simulink model "Demo_IntelligentTrafficSigns_cs"  (Simulink 7.6)
settings: Solver=FixedStepDiscrete  SolverMode=SingleTasking  StartTime=0.0  StopTime=66  FixedStep=1/10  RelTol=1e-3
PreLoadFcn:
  | check_NUTS_defined
  | 
  | assignin('base',['model_closed_' bdroot],false);
InitFcn:
  | PreScanExperimentInitFcn(bdroot)
StopFcn:
  | PreScanExperimentStopFcn(bdroot);
  | 
undefined symbols (not defined in the file or in its folder): DiscIntNeverNeededParam, magnitude, permission, R_curve, Results, Results_20090205_104801, spare_10, spare_4, spare_5, spare_6, spare_7, spare_8, spare_9, velocity_limit, x_ini_VRML, y_ini_VRML, z_ini_VRML
libraries: simulink_extras x8, PreScanSimulinkLibrary x7, simulink x5, PreScanUsefulBlocks x3
(part 9 of 10)

== system Demo_IntelligentTrafficSigns_cs/VISUWheels_BMW_X5_1/conv_RotWheelRR ==
  BryantISO [deg] : Inport
  Fcn10 : Fcn  Expr=cos(u(1))*sin(u(3))+sin(u(1))*sin(u(2))*cos(u(3))
  Fcn11 : Fcn  Expr=sin(u(1))*sin(u(3))-cos(u(1))*sin(u(2))*cos(u(3))
  Fcn12 : Fcn  Expr=-cos(u(2))*sin(u(3))
  Fcn13 : Fcn  Expr=cos(u(1))*cos(u(3))-sin(u(1))*sin(u(2))*sin(u(3))
  Fcn14 : Fcn  Expr=sin(u(1))*cos(u(3))+cos(u(1))*sin(u(2))*sin(u(3))
  Fcn15 : Fcn  Expr=sin(u(2))
  Fcn16 : Fcn  Expr=-sin(u(1))*cos(u(2))
  Fcn17 : Fcn  Expr=cos(u(1))*cos(u(2))
  Fcn9 : Fcn  Expr=cos(u(2))*cos(u(3))
  Mux1 : Mux  Inputs=9
  RotMat2AxisAngle : Subsystem
  deg2rad : Gain  Gain=pi/180
  xyz 2 zxy : Selector  InputPortWidth=3  IndexOptions=Index vector (dialog)  Indices=[3 1 2]  OutputSizes=1
  zxy 2 xyz : Selector  InputPortWidth=4  IndexOptions=Index vector (dialog)  Indices=[2 3 1 4]  OutputSizes=1
  QuaternionVisu [rad] : Outport
  wires:
  zxy 2 xyz/1 -> QuaternionVisu [rad]/1
  xyz 2 zxy/1 -> deg2rad/1
  deg2rad/1 -> Fcn17/1, Fcn16/1, Fcn15/1, Fcn14/1, Fcn13/1, Fcn12/1, Fcn11/1, Fcn10/1, Fcn9/1
  BryantISO [deg]/1 -> xyz 2 zxy/1  "BryantAngles rotx roty rotz Visu"
  RotMat2AxisAngle/1(Angles_Visu) -> zxy 2 xyz/1
  Mux1/1 -> RotMat2AxisAngle/1(RotMat_Visu)  "RotationMatrix Visu"
  Fcn17/1 -> Mux1/9
  Fcn16/1 -> Mux1/8
  Fcn15/1 -> Mux1/7
  Fcn14/1 -> Mux1/6
  Fcn13/1 -> Mux1/5
  Fcn12/1 -> Mux1/4
  Fcn11/1 -> Mux1/3
  Fcn10/1 -> Mux1/2
  Fcn9/1 -> Mux1/1

== system Demo_IntelligentTrafficSigns_cs/VISUWheels_BMW_X5_1/conv_TransWheelFL ==
  transISO [m] : Inport
  Gain1 : Gain  Gain=Gain_xISO
  Gain2 : Gain  Gain=Gain_yISO
  Gain3 : Gain  Gain=Gain_zISO
  Mux2 : Mux  Inputs=3
  Sum : Sum  Inputs=|++
  pos_ini_Visu : Subsystem
  transISO2Visu : Subsystem
  transVisu [m] : Outport
  wires:
  Gain3/1 -> Mux2/3
  Gain2/1 -> Mux2/2
  Gain1/1 -> Mux2/1
  Sum/1 -> transVisu [m]/1
  pos_ini_Visu/1(pos_ini_Visu) -> Sum/2
  Mux2/1 -> Sum/1
  transISO [m]/1 -> transISO2Visu/1(transISO)
  transISO2Visu/1(trans_x_Visu) -> Gain1/1
  transISO2Visu/2(trans_y_Visu) -> Gain2/1
  transISO2Visu/3(trans_z_Visu) -> Gain3/1

== system Demo_IntelligentTrafficSigns_cs/VISUWheels_BMW_X5_1/conv_TransWheelFR ==
  transISO [m] : Inport
  Gain1 : Gain  Gain=Gain_xISO
  Gain2 : Gain  Gain=Gain_yISO
  Gain3 : Gain  Gain=Gain_zISO
  Mux2 : Mux  Inputs=3
  Sum : Sum  Inputs=|++
  pos_ini_Visu : Subsystem
  transISO2Visu : Subsystem
  transVisu [m] : Outport
  wires:
  Gain3/1 -> Mux2/3
  Gain2/1 -> Mux2/2
  Gain1/1 -> Mux2/1
  Sum/1 -> transVisu [m]/1
  pos_ini_Visu/1(pos_ini_Visu) -> Sum/2
  Mux2/1 -> Sum/1
  transISO [m]/1 -> transISO2Visu/1(transISO)
  transISO2Visu/1(trans_x_Visu) -> Gain1/1
  transISO2Visu/2(trans_y_Visu) -> Gain2/1
  transISO2Visu/3(trans_z_Visu) -> Gain3/1

== system Demo_IntelligentTrafficSigns_cs/VISUWheels_BMW_X5_1/conv_TransWheelRL ==
  transISO [m] : Inport
  Gain1 : Gain  Gain=Gain_xISO
  Gain2 : Gain  Gain=Gain_yISO
  Gain3 : Gain  Gain=Gain_zISO
  Mux2 : Mux  Inputs=3
  Sum : Sum  Inputs=|++
  pos_ini_Visu : Subsystem
  transISO2Visu : Subsystem
  transVisu [m] : Outport
  wires:
  Gain3/1 -> Mux2/3
  Gain2/1 -> Mux2/2
  Gain1/1 -> Mux2/1
  Sum/1 -> transVisu [m]/1
  pos_ini_Visu/1(pos_ini_Visu) -> Sum/2
  Mux2/1 -> Sum/1
  transISO2Visu/3(trans_z_Visu) -> Gain3/1
  transISO2Visu/2(trans_y_Visu) -> Gain2/1
  transISO [m]/1 -> transISO2Visu/1(transISO)
  transISO2Visu/1(trans_x_Visu) -> Gain1/1

== system Demo_IntelligentTrafficSigns_cs/VISUWheels_BMW_X5_1/conv_TransWheelRR ==
  transISO [m] : Inport
  Gain1 : Gain  Gain=Gain_xISO
  Gain2 : Gain  Gain=Gain_yISO
  Gain3 : Gain  Gain=Gain_zISO
  Mux2 : Mux  Inputs=3
  Sum : Sum  Inputs=|++
  pos_ini_Visu : Subsystem
  transISO2Visu : Subsystem
  transVisu [m] : Outport
  wires:
  Gain3/1 -> Mux2/3
  Gain2/1 -> Mux2/2
  Gain1/1 -> Mux2/1
  Sum/1 -> transVisu [m]/1
  pos_ini_Visu/1(pos_ini_Visu) -> Sum/2
  Mux2/1 -> Sum/1
  transISO2Visu/3(trans_z_Visu) -> Gain3/1
  transISO2Visu/2(trans_y_Visu) -> Gain2/1
  transISO [m]/1 -> transISO2Visu/1(transISO)
  transISO2Visu/1(trans_x_Visu) -> Gain1/1

== system Demo_IntelligentTrafficSigns_cs/VISUWheels_BMW_X5_1/visu_RotWheelFL ==
  Y : Inport
  Assignment : Assignment  IndexMode=Zero-based  SampleTime=TimeStep  IndexOptions=Starting index (dialog)  Indices=PortIndex  OutputSizes=[1 1]
  Data Store Read : DataStoreRead  DataStoreName=SimCoreActuators  SampleTime=TimeStep
  Data Store Write : DataStoreWrite  DataStoreName=SimCoreActuators
  wires:
  Y/1 -> Assignment/2
  Data Store Read/1 -> Assignment/1
  Assignment/1 -> Data Store Write/1

== system Demo_IntelligentTrafficSigns_cs/VISUWheels_BMW_X5_1/visu_RotWheelFR ==
  Y : Inport
  Assignment : Assignment  IndexMode=Zero-based  SampleTime=TimeStep  IndexOptions=Starting index (dialog)  Indices=PortIndex  OutputSizes=[1 1]
  Data Store Read : DataStoreRead  DataStoreName=SimCoreActuators  SampleTime=TimeStep
  Data Store Write : DataStoreWrite  DataStoreName=SimCoreActuators
  wires:
  Y/1 -> Assignment/2
  Data Store Read/1 -> Assignment/1
  Assignment/1 -> Data Store Write/1

== system Demo_IntelligentTrafficSigns_cs/VISUWheels_BMW_X5_1/visu_RotWheelRL ==
  Y : Inport
  Assignment : Assignment  IndexMode=Zero-based  SampleTime=TimeStep  IndexOptions=Starting index (dialog)  Indices=PortIndex  OutputSizes=[1 1]
  Data Store Read : DataStoreRead  DataStoreName=SimCoreActuators  SampleTime=TimeStep
  Data Store Write : DataStoreWrite  DataStoreName=SimCoreActuators
  wires:
  Y/1 -> Assignment/2
  Data Store Read/1 -> Assignment/1
  Assignment/1 -> Data Store Write/1

== system Demo_IntelligentTrafficSigns_cs/VISUWheels_BMW_X5_1/visu_RotWheelRR ==
  Y : Inport
  Assignment : Assignment  IndexMode=Zero-based  SampleTime=TimeStep  IndexOptions=Starting index (dialog)  Indices=PortIndex  OutputSizes=[1 1]
  Data Store Read : DataStoreRead  DataStoreName=SimCoreActuators  SampleTime=TimeStep
  Data Store Write : DataStoreWrite  DataStoreName=SimCoreActuators
  wires:
  Y/1 -> Assignment/2
  Data Store Read/1 -> Assignment/1
  Assignment/1 -> Data Store Write/1

== system Demo_IntelligentTrafficSigns_cs/VISUWheels_BMW_X5_1/visu_TransWheelFL ==
  Y : Inport
  Assignment : Assignment  IndexMode=Zero-based  SampleTime=TimeStep  IndexOptions=Starting index (dialog)  Indices=PortIndex  OutputSizes=[1 1]
  Data Store Read : DataStoreRead  DataStoreName=SimCoreActuators  SampleTime=TimeStep
  Data Store Write : DataStoreWrite  DataStoreName=SimCoreActuators
  wires:
  Y/1 -> Assignment/2
  Data Store Read/1 -> Assignment/1
  Assignment/1 -> Data Store Write/1

== system Demo_IntelligentTrafficSigns_cs/VISUWheels_BMW_X5_1/visu_TransWheelFR ==
  Y : Inport
  Assignment : Assignment  IndexMode=Zero-based  SampleTime=TimeStep  IndexOptions=Starting index (dialog)  Indices=PortIndex  OutputSizes=[1 1]
  Data Store Read : DataStoreRead  DataStoreName=SimCoreActuators  SampleTime=TimeStep
  Data Store Write : DataStoreWrite  DataStoreName=SimCoreActuators
  wires:
  Y/1 -> Assignment/2
  Data Store Read/1 -> Assignment/1
  Assignment/1 -> Data Store Write/1

== system Demo_IntelligentTrafficSigns_cs/VISUWheels_BMW_X5_1/visu_TransWheelRL ==
  Y : Inport
  Assignment : Assignment  IndexMode=Zero-based  SampleTime=TimeStep  IndexOptions=Starting index (dialog)  Indices=PortIndex  OutputSizes=[1 1]
  Data Store Read : DataStoreRead  DataStoreName=SimCoreActuators  SampleTime=TimeStep
  Data Store Write : DataStoreWrite  DataStoreName=SimCoreActuators
  wires:
  Y/1 -> Assignment/2
  Data Store Read/1 -> Assignment/1
  Assignment/1 -> Data Store Write/1

== system Demo_IntelligentTrafficSigns_cs/VISUWheels_BMW_X5_1/visu_TransWheelRR ==
  Y : Inport
  Assignment : Assignment  IndexMode=Zero-based  SampleTime=TimeStep  IndexOptions=Starting index (dialog)  Indices=PortIndex  OutputSizes=[1 1]
  Data Store Read : DataStoreRead  DataStoreName=SimCoreActuators  SampleTime=TimeStep
  Data Store Write : DataStoreWrite  DataStoreName=SimCoreActuators
  wires:
  Y/1 -> Assignment/2
  Data Store Read/1 -> Assignment/1
  Assignment/1 -> Data Store Write/1

== system Demo_IntelligentTrafficSigns_cs/BMW_X5_1/ANIMATION PORTS Tuning/Detect Decrease ==
  U : Inport
  Constant1 : Constant  Value=0.1
  Data Type Conversion : DataTypeConversion  OutDataTypeStr=double
  Delay Input1 : UnitDelay  Description=Store in Global RAM  X0=vinit  SampleTime=-1
  Relational Operator : RelationalOperator  OutDataTypeStr=boolean
  Sum : Sum  Inputs=|-+
  Y : Outport  InitialOutput=0
  wires:
  U/1 -> Delay Input1/1, Sum/1  "U(k)"
  Delay Input1/1 -> Sum/2  "U(k-1)"
  Sum/1 -> Relational Operator/1  "Edge"
  Relational Operator/1 -> Data Type Conversion/1
  Constant1/1 -> Relational Operator/2
  Data Type Conversion/1 -> Y/1

== system Demo_IntelligentTrafficSigns_cs/BMW_X5_1/ANIMATION PORTS Tuning/Subsystem ==
  state : Inport
  Discrete Derivative : simulink/Discrete/Discrete Derivative  SystemSampleTime=-1  gainval=1.0  ICPrevScaledInput=0.0  OutMin=[]  OutMax=[]  OutDataType=fixdt(1, 16)  OutScaling=2^-10  DoSatur=off
  Gain4 : Gain
  Gain5 : Gain  Gain=0.25
  Radians to Degrees : simulink_extras/Transformations/Radians to Degrees  SystemSampleTime=-1
  Selector1 : Selector  InputPortWidth=9  IndexOptions=Index vector (dialog)  Indices=9  OutputSizes=1
  Steer [deg] : Outport
  Steer Angle Front [deg] : Outport  Port=2
  wires:
  Selector1/1 -> Radians to Degrees/1
  Radians to Degrees/1 -> Discrete Derivative/1
  Discrete Derivative/1 -> Gain4/1, Gain5/1
  state/1 -> Selector1/1
  Gain4/1 -> Steer [deg]/1
  Gain5/1 -> Steer Angle Front [deg]/1

== system Demo_IntelligentTrafficSigns_cs/BMW_X5_1/Car_Rx_1_Demux/Reorder ==
  In : Inport
  Selector1 : Selector  InputPortWidth=InputPortWidth  IndexOptions=Index vector (dialog)  Indices=Elements  OutputSizes=1
  Out : Outport
  wires:
  Selector1/1 -> Out/1
  In/1 -> Selector1/1

== system Demo_IntelligentTrafficSigns_cs/BMW_X5_1/Car_Rx_1_Demux/compas converter ==
  rotz [rad] : Inport
  cart2compas : Subsystem
  rad2deg : Gain  Gain=180/pi
  heading [deg] : Outport
  wires:
  rad2deg/1 -> cart2compas/1(Angle [deg])
  rotz [rad]/1 -> rad2deg/1
  cart2compas/1(heading [deg]) -> heading [deg]/1

== system Demo_IntelligentTrafficSigns_cs/BMW_X5_1/INTERPRETATION ALGORITHM TrafficSign Intelligence/Convert and Sort Sensor Data ==
  ids [-] : Inport
  lats [deg] : Inport  Port=2
  long [deg] : Inport  Port=3
  alts [m] : Inport  Port=4
  headings [deg] : Inport  Port=5
  speed_limits [km/h] : Inport  Port=6
  notifications [-] : Inport  Port=7
  R_curves [m] : Inport  Port=8
  Demux1 : Demux  Outputs=3
  GPS2xyz : M-S-Function  FunctionName=msfcn_GPS2xyz  Parameters=5 GPS_base  mask{GPS_base=[51.475517 5.626375 0.000]}
      mask prompts: GPS_base "GPS coordinate of the origin of the xyz coordinate system [lat lon alt]:"
      mask help:
      | Converts GPS coordinates to local xyz coordinates.
      |
      | The xyz coordinate system is defined as the tangential plane at the specified GPS coordinate (to be defined below), with x pointing east, y pointing north and z pointing to the outside of the earth.
      mask Display:
      | port_label('input', 1, 'Lat,Long,Alt [deg,deg,m]')
      | port_label('output', 1, 'x,y,z [m]')
  Mux : Mux  Inputs=3
  Sort Output Based on ID : Subsystem  mask(Sort output){mode=descending, portdim=5}
      mask prompts: mode "Sorting mode"; portdim "Port dimension"
      mask help: This function sorts the input in ascending or descending order and outputs the an index array of the output order.
      mask init:
      | switch mode
      |     case 1
      |         OutputOrder=[1:1:portdim];
      |     case 2
      |         OutputOrder=[portdim:-1:1];
      | end
      mask StyleString: popup(ascending|descending),edit
      mask Display: port_label('input',1,'sort_input')
  Terminator2 : Terminator
  Terminator7 : Terminator
  Terminator8 : Terminator
  ids  [-] : Outport
  headings  [deg] : Outport  Port=2
  xs  [m] : Outport  Port=3
  ys [m] : Outport  Port=4
  velocity_limits  [km/h] : Outport  Port=5
  notifications  [-] : Outport  Port=6
  R_curves  [m] : Outport  Port=7
  wires:
  Sort Output Based on ID/9(R_curves  [m]) -> R_curves  [m]/1
  R_curves [m]/1 -> Sort Output Based on ID/10(R_curves [m])
  Sort Output Based on ID/8(notifications  [-]) -> notifications  [-]/1
  notifications [-]/1 -> Sort Output Based on ID/9(notifications [-])
  Sort Output Based on ID/7(velocity_limits  [km/h]) -> velocity_limits  [km/h]/1
  speed_limits [km/h]/1 -> Sort Output Based on ID/8(velocity_limits [km/h])
  headings [deg]/1 -> Sort Output Based on ID/7(headings [deg])
  Sort Output Based on ID/6(ys  [m]) -> ys [m]/1
  alts [m]/1 -> Mux/3
  Sort Output Based on ID/5(xs  [m]) -> xs  [m]/1
  Sort Output Based on ID/4(headings  [deg]) -> headings  [deg]/1
  Sort Output Based on ID/1(ids  [-]) -> ids  [-]/1
  Sort Output Based on ID/2(lats  [deg]) -> Terminator7/1
  Sort Output Based on ID/3(longs  [deg]) -> Terminator8/1  "heading_objects [deg]"
  GPS2xyz/1 -> Demux1/1
  Demux1/1 -> Sort Output Based on ID/5(xs [m])
  Demux1/2 -> Sort Output Based on ID/6(ys [m])
  Demux1/3 -> Terminator2/1
  Mux/1 -> GPS2xyz/1
  long [deg]/1 -> Mux/2, Sort Output Based on ID/4(longs [deg])
  lats [deg]/1 -> Mux/1, Sort Output Based on ID/3(lats [deg])
  ids [-]/1 -> Sort Output Based on ID/1(..), Sort Output Based on ID/2(ids [-])

== system Demo_IntelligentTrafficSigns_cs/BMW_X5_1/INTERPRETATION ALGORITHM TrafficSign Intelligence/NOTIFICATION  UPCOMING TRAFFIC SIGN ==
  notifications [-] : Inport
  velocity_limits [km/h] : Inport  Port=2
  traffic sign ids [-] : Inport  Port=3
  HOLD logic : Subsystem
  Product3 : Product  RndMeth=Zero
  Retrieve upcoming prescribed velocity : Subsystem
  upcoming speed limit [km/h] : Outport
  traffic sign ids  [-] : Outport  Port=2
  wires:
  traffic sign ids [-]/1 -> Product3/2
  velocity_limits [km/h]/1 -> Retrieve upcoming prescribed velocity/2(velocity_limits [km/h])
  notifications [-]/1 -> Product3/1, Retrieve upcoming prescribed velocity/1(notifications [-])
  Retrieve upcoming prescribed velocity/1(upcoming velocity_limit  [km/h]) -> HOLD logic/1(In)
  HOLD logic/1(Out) -> upcoming speed limit [km/h]/1
  Product3/1 -> traffic sign ids  [-]/1

== system Demo_IntelligentTrafficSigns_cs/BMW_X5_1/INTERPRETATION ALGORITHM TrafficSign Intelligence/RECOMMENDED  SAFE SPEED IN CURVE ==
  note: unloaded
  note: loaded
  note: ay LIMIT
  notifications [-] : Inport
  R_curves [-] : Inport  Port=2
  velocity [m/s] : Inport  Port=3
  Constant : Constant  Value=3
  Constant1 : Constant  Value=5
  Curve warning mechanism define recommende speed based on host_velocity and curve radius : Subsystem
  Gain1 : Gain  Gain=3.6
  HOLD logic1 : Subsystem
  Manual Switch : simulink/Signal Routing/Manual Switch  SystemSampleTime=-1  sw=1  action=0  varsize=off
  Replace zero radius (no warning) with infinite radius : Subsystem
  Retrieve radius of upcoming curve : Subsystem
  Terminator : Terminator
  Terminator1 : Terminator
  Terminator2 : Terminator
  vmax : Saturate  UpperLimit=999  LowerLimit=0
  recommended  speed  in curve [km/h] : Outport
  wires:
  Gain1/1 -> vmax/1
  Curve warning mechanism define recommende speed based on host_velocity and curve radius/4(R_safe [m]) -> Terminator1/1
  Curve warning mechanism define recommende speed based on host_velocity and curve radius/3(ay_limit [m/s2]) -> Terminator/1
  Curve warning mechanism define recommende speed based on host_velocity and curve radius/2(warning [-]) -> Terminator2/1
  Curve warning mechanism define recommende speed based on host_velocity and curve radius/1(v_recommended [m/s]) -> Gain1/1
  Replace zero radius (no warning) with infinite radius/1(filt_R_curve [m]) -> Curve warning mechanism define recommende speed based on host_velocity and curve radius/2(R_curve [m])
  notifications [-]/1 -> Retrieve radius of upcoming curve/1(notifications [-])
  R_curves [-]/1 -> Retrieve radius of upcoming curve/2(R_curves [m])
  velocity [m/s]/1 -> Curve warning mechanism define recommende speed based on host_velocity and curve radius/1(velocity [m/s])
  vmax/1 -> recommended  speed  in curve [km/h]/1
  Retrieve radius of upcoming curve/1(R_curves   [m]) -> HOLD logic1/1(In)
  Constant/1 -> Manual Switch/2
  Constant1/1 -> Manual Switch/1
  Manual Switch/1 -> Curve warning mechanism define recommende speed based on host_velocity and curve radius/3(allowed ay limit [m/s2])
  HOLD logic1/1(Out) -> Replace zero radius (no warning) with infinite radius/1(R_curve [m])

== system Demo_IntelligentTrafficSigns_cs/BMW_X5_1/INTERPRETATION ALGORITHM TrafficSign Intelligence/SPEED LIMIT ==
  permissions [-] : Inport
  velocity_limits [km/h] : Inport  Port=2
  HOLD logic : Subsystem
  Retrieve Prescribed Velocity : Subsystem
  velocity_limit [km/h] : Outport
  wires:
  permissions [-]/1 -> Retrieve Prescribed Velocity/1(permissions [-])
  HOLD logic/1(Out) -> velocity_limit [km/h]/1
  velocity_limits [km/h]/1 -> Retrieve Prescribed Velocity/2(velocity_limits [km/h])
  Retrieve Prescribed Velocity/1(velocity_limit [km/h]) -> HOLD logic/1(In)

== system Demo_IntelligentTrafficSigns_cs/BMW_X5_1/INTERPRETATION ALGORITHM TrafficSign Intelligence/TRAFFIC SIGN DETECTION based on relative  position and orientation ==
  note: Driver will be NOTIFIED of a traffic sign
      | in case the traffic sign is within
      | - x-range of 0 to 42 meters ahead
      | - y-range -2 to 5 meters to the right of vehicle
  note: Traffic sign is relevant in case its sign plate
      | is headed (within a range of +/- 45 deg)
      | towards the approaching vehicle
  note: The traffic sign rule become a PERMIT (valid/obligitory)
      | when driver passes the traffic sign in the following range:
      | - x-range of 0 to 14 meters ahead
      | - y-range -2 to 5 meters to the right of vehicle
      |
      | (e.g. speed limit become a permit)
  x_host [m] : Inport
  y_host [m] : Inport  Port=2
  heading_host [deg] : Inport  Port=3
  headings [deg] : Inport  Port=4
  xs [m] : Inport  Port=5
  ys [m] : Inport  Port=6
  Product1 : Product  RndMeth=Zero
  Product2 : Product  RndMeth=Zero
  TRAFFIC SIGN DETECTION: based on Heading : Subsystem
  TRAFFIC SIGN DETECTION: based on Position : Subsystem
  permmissions [-] : Outport
  notifications [-] : Outport  Port=2
  wires:
  xs [m]/1 -> TRAFFIC SIGN DETECTION: based on Position/3(xs [m])
  ys [m]/1 -> TRAFFIC SIGN DETECTION: based on Position/4(ys [m])
  heading_host [deg]/1 -> TRAFFIC SIGN DETECTION: based on Position/5(heading_host [deg]), TRAFFIC SIGN DETECTION: based on Heading/1(heading_host [deg])
  x_host [m]/1 -> TRAFFIC SIGN DETECTION: based on Position/1(x_host [m])
  y_host [m]/1 -> TRAFFIC SIGN DETECTION: based on Position/2(y_host [m])
  TRAFFIC SIGN DETECTION: based on Position/1(permissions [-]) -> Product2/1
  TRAFFIC SIGN DETECTION: based on Heading/1(relevance [-]) -> Product1/2, Product2/2
  headings [deg]/1 -> TRAFFIC SIGN DETECTION: based on Heading/2(headings [deg])
  TRAFFIC SIGN DETECTION: based on Position/2(notifications [-]) -> Product1/1
  Product1/1 -> notifications [-]/1
  Product2/1 -> permmissions [-]/1

== system Demo_IntelligentTrafficSigns_cs/BMW_X5_1/INTERPRETATION ALGORITHM TrafficSign Intelligence/VELOCITY REDUCTION  CTRL ==
  velocity [km/h/] : Inport
  speed_limit [km/h] : Inport  Port=2
  curve_velocity [km/h] : Inport  Port=3
  Discrete-Time Integrator : DiscreteIntegrator  SampleTime=1/10  ICPrevOutput=DiscIntNeverNeededParam  ICPrevScaledInput=DiscIntNeverNeededParam
  Discrete-Time Integrator1 : DiscreteIntegrator  gainval=0.01  SampleTime=1/10  ICPrevOutput=DiscIntNeverNeededParam  ICPrevScaledInput=DiscIntNeverNeededParam
  Gain1 : Gain  Gain=1/3.6
  Gain2 : Gain  Gain=1.5
  Gain3 : Gain  Gain=1/3.6
  MinMax : MinMax  Inputs=2
  Sum1 : Sum  Inputs=|++
  Sum2 : Sum  Inputs=|+-
  delta_v [m/s] : Outport
  wires:
  velocity [km/h/]/1 -> Gain1/1
  Sum2/1 -> Gain2/1, Discrete-Time Integrator1/1
  Discrete-Time Integrator/1 -> delta_v [m/s]/1
  Gain3/1 -> Sum2/2
  MinMax/1 -> Gain3/1
  Sum1/1 -> Discrete-Time Integrator/1
  Gain1/1 -> Sum2/1
  speed_limit [km/h]/1 -> MinMax/1
  curve_velocity [km/h]/1 -> MinMax/2
  Gain2/1 -> Sum1/1
  Discrete-Time Integrator1/1 -> Sum1/2

== system Demo_IntelligentTrafficSigns_cs/BMW_X5_1/SELF_Demux/compas converter ==
  rotz [rad] : Inport
  cart2compas : Subsystem
  rad2deg : Gain  Gain=180/pi
  heading [deg] : Outport
  wires:
  cart2compas/1(heading [deg]) -> heading [deg]/1
  rotz [rad]/1 -> rad2deg/1
  rad2deg/1 -> cart2compas/1(Angle [deg])

== system Demo_IntelligentTrafficSigns_cs/BMW_X5_1/Trajectories/Add Roll & Pitch Behaviour ==
  State_In : Inport
  Sum : Sum  Inputs=+++
  calculate Pitch Angle : Subsystem
  calculate Roll Angle : Subsystem
  State_Out : Outport
  wires:
  State_In/1 -> Sum/1, calculate Roll Angle/1(In1), calculate Pitch Angle/1(In1)
  calculate Roll Angle/1(Out1) -> Sum/2
  Sum/1 -> State_Out/1
  calculate Pitch Angle/1(Out1) -> Sum/3

== system Demo_IntelligentTrafficSigns_cs/BMW_X5_1/Trajectories/ElapsedTrack1 ==
  Offset : Inport
  s : Inport  Port=2
  Mux : Mux  Inputs=3
  Mux1 : Mux  Inputs=3
  MuxState : Subsystem
  OffsetCor : Subsystem
  pitchAngle : Lookup  InputValues=s  Table=pitch  LookUpMeth=Interpolation-Use End Values
  rollAngle : Lookup  InputValues=s  Table=roll  LookUpMeth=Interpolation-Use End Values
  xpos : Lookup  InputValues=s  Table=x  LookUpMeth=Interpolation-Use End Values
  yawAngle : Lookup  InputValues=s  Table=yaw  LookUpMeth=Interpolation-Use End Values
  ypos : Lookup  InputValues=s  Table=y  LookUpMeth=Interpolation-Use End Values
  zpos : Lookup  InputValues=s  Table=z  LookUpMeth=Interpolation-Use End Values
  state : Outport
  wires:
  zpos/1 -> OffsetCor/3(z pos)
  rollAngle/1 -> OffsetCor/4(R angle)
  pitchAngle/1 -> OffsetCor/5(P angle)
  yawAngle/1 -> OffsetCor/6(Y angle)
  OffsetCor/6(Y angle_cor) -> Mux1/3
  OffsetCor/5(P angle_cor) -> Mux1/2
  OffsetCor/4(R angle_cor) -> Mux1/1
  OffsetCor/3(z pos_cor) -> Mux/3
  MuxState/1(State) -> state/1
  Mux/1 -> MuxState/1(xyzPos_[m])
  s/1 -> pitchAngle/1, yawAngle/1, rollAngle/1, zpos/1, ypos/1, xpos/1
  Offset/1 -> OffsetCor/7(Offset)
  xpos/1 -> OffsetCor/1(x pos)
  ypos/1 -> OffsetCor/2(y pos)
  OffsetCor/1(x pos_cor) -> Mux/1
  OffsetCor/2(y pos_cor) -> Mux/2
  Mux1/1 -> MuxState/2(RPYAngle_[rad])

== system Demo_IntelligentTrafficSigns_cs/BMW_X5_1/Trajectories/PathFollowerSva_1 ==
  From Workspace : FromWorkspace  VariableName=trajectory  SampleTime=TimeStep  OutputAfterFinalValue=Holding final value
  From Workspace1 : FromWorkspace  VariableName=trajectory  SampleTime=TimeStep  OutputAfterFinalValue=Setting to zero
  Mux : Mux  Inputs=2
  Selector1 : Selector  InputPortWidth=24  IndexOptions=Index vector (dialog)  Indices=[7:18]  OutputSizes=1
  Selector9 : Selector  InputPortWidth=24  IndexOptions=Index vector (dialog)  Indices=[1:6]  OutputSizes=1
  trajectory : Outport
  wires:
  Selector1/1 -> Mux/2
  Selector9/1 -> Mux/1
  Mux/1 -> trajectory/1
  From Workspace1/1 -> Selector1/1
  From Workspace/1 -> Selector9/1

== system Demo_IntelligentTrafficSigns_cs/BMW_X5_1/Trajectories/Traj2State1 ==
  Traj Org : Inport
  Offset : Inport  Port=2
  Add2 : Sum
  ApplyRotation : PreScanSimulinkLibrary/ApplyRotation  SystemSampleTime=-1
  ApplyRotation1 : PreScanSimulinkLibrary/ApplyRotation  SystemSampleTime=-1
  ApplyRotation2 : PreScanSimulinkLibrary/ApplyRotation  SystemSampleTime=-1
  Constant : Constant  Value=zeros(12,1)
  Mux : Mux  Inputs=6
  Mux1 : Mux  Inputs=2
  Mux{2,4..9} (7 alike) : Mux  Inputs=3
  Mux3 : Mux  Inputs=2
  Selector : Selector  InputPortWidth=6  IndexOptions=Index vector (dialog)  Indices=[4 5 6]  OutputSizes=1
  Selector1 : Selector  InputPortWidth=21  IndexOptions=Index vector (dialog)  Indices=[5]  OutputSizes=1
  Selector10 : Selector  InputPortWidth=21  IndexOptions=Index vector (dialog)  Indices=[2]  OutputSizes=1
  Selector11 : Selector  InputPortWidth=21  IndexOptions=Index vector (dialog)  Indices=[3]  OutputSizes=1
  Selector12 : Selector  InputPortWidth=21  IndexOptions=Index vector (dialog)  Indices=[1]  OutputSizes=1
  Selector13 : Selector  InputPortWidth=21  IndexOptions=Index vector (dialog)  Indices=[8]  OutputSizes=1
  Selector14 : Selector  InputPortWidth=21  IndexOptions=Index vector (dialog)  Indices=[9]  OutputSizes=1
  Selector15 : Selector  InputPortWidth=21  IndexOptions=Index vector (dialog)  Indices=[7]  OutputSizes=1
  Selector16 : Selector  InputPortWidth=21  IndexOptions=Index vector (dialog)  Indices=[14]  OutputSizes=1
  Selector17 : Selector  InputPortWidth=21  IndexOptions=Index vector (dialog)  Indices=[15]  OutputSizes=1
  Selector18 : Selector  InputPortWidth=21  IndexOptions=Index vector (dialog)  Indices=[13]  OutputSizes=1
  Selector19 : Selector  InputPortWidth=21  IndexOptions=Index vector (dialog)  Indices=[20]  OutputSizes=1
  Selector2 : Selector  InputPortWidth=21  IndexOptions=Index vector (dialog)  Indices=[6]  OutputSizes=1
  Selector20 : Selector  InputPortWidth=21  IndexOptions=Index vector (dialog)  Indices=[21]  OutputSizes=1
  Selector21 : Selector  InputPortWidth=21  IndexOptions=Index vector (dialog)  Indices=[19]  OutputSizes=1
  Selector3 : Selector  InputPortWidth=21  IndexOptions=Index vector (dialog)  Indices=[12]  OutputSizes=1
  Selector4 : Selector  InputPortWidth=21  IndexOptions=Index vector (dialog)  Indices=[17]  OutputSizes=1
  Selector5 : Selector  InputPortWidth=21  IndexOptions=Index vector (dialog)  Indices=[18]  OutputSizes=1
  Selector6 : Selector  InputPortWidth=21  IndexOptions=Index vector (dialog)  Indices=[16]  OutputSizes=1
  Selector7 : Selector  InputPortWidth=21  IndexOptions=Index vector (dialog)  Indices=[11]  OutputSizes=1
  Selector8 : Selector  InputPortWidth=21  IndexOptions=Index vector (dialog)  Indices=[10]  OutputSizes=1
  Selector9 : Selector  InputPortWidth=21  IndexOptions=Index vector (dialog)  Indices=[4]  OutputSizes=1
  Traj2State2 : Subsystem
  state : Outport
  wires:
  Mux9/1 -> ApplyRotation2/2, ApplyRotation1/2, ApplyRotation/2
  Selector20/1 -> Mux9/3
  Selector19/1 -> Mux9/2
  Selector21/1 -> Mux9/1
  Selector5/1 -> Mux8/3
  Selector4/1 -> Mux8/2
  Selector6/1 -> Mux8/1
  Mux8/1 -> Mux/6
  Mux6/1 -> ApplyRotation2/1
  Mux7/1 -> Mux/4
  ApplyRotation2/1 -> Mux/5
  Selector3/1 -> Mux7/3
  Selector7/1 -> Mux7/2
  Selector8/1 -> Mux7/1
  Mux4/1 -> Mux/2
  Mux5/1 -> ApplyRotation1/1
  ApplyRotation1/1 -> Mux/3
  Mux2/1 -> ApplyRotation/1
  ApplyRotation/1 -> Mux/1
  Selector2/1 -> Mux4/3
  Selector1/1 -> Mux4/2
  Selector9/1 -> Mux4/1
  Selector17/1 -> Mux6/3
  Selector16/1 -> Mux6/2
  Selector18/1 -> Mux6/1
  Selector14/1 -> Mux5/3
  Selector13/1 -> Mux5/2
  Selector15/1 -> Mux5/1
  Selector11/1 -> Mux2/3
  Selector10/1 -> Mux2/2
  Selector12/1 -> Mux2/1
  Mux3/1 -> Selector8/1, Selector7/1, Selector3/1, Selector18/1, Selector16/1, Selector17/1, Selector6/1, Selector20/1, Selector19/1, Selector21/1, Selector5/1, Selector4/1, Selector14/1, Selector13/1, Selector15/1, Selector2/1, Selector1/1, Selector9/1, Selector12/1, Selector10/1, Selector11/1
  Mux1/1 -> Add2/2
  Constant/1 -> Mux1/2
  Add2/1 -> Traj2State2/1(traj)
  Selector/1 -> Mux3/2
  Traj Org/1 -> Mux3/1
  Offset/1 -> Mux1/1, Selector/1
  Mux/1 -> Add2/1
  Traj2State2/1(state) -> state/1

== system Demo_IntelligentTrafficSigns_cs/CameraTrolley/Dynamics_Empty/MuxState ==
  note: mode: Dynamics
  note: mode: Positioning
  note: mode: Dynamics
      | VelVEHICLE
      | wrt VEH
  note: AnglesVEHICLE
      | wrtGLOB
  note: For state mode 'Dynamic' positions
      | are not taken into account, as they
      | are computed by integrating velocity.
      | Click annotation for Help.
  x [m] : Inport  SampleTime=TimeStep
  y [m] : Inport  Port=2  SampleTime=TimeStep
  z [m] : Inport  Port=3  SampleTime=TimeStep
  dx [m/s] : Inport  Port=4  SampleTime=TimeStep
  dy [m/s] : Inport  Port=5  SampleTime=TimeStep
  dz [m/s] : Inport  Port=6  SampleTime=TimeStep
  R [rad] : Inport  Port=7  SampleTime=TimeStep
  P [rad] : Inport  Port=8  SampleTime=TimeStep
  Y [rad] : Inport  Port=9  SampleTime=TimeStep
  Constant : Constant  DisableCoverage=on  Value=positioningmode
  Discrete-Time Integrator : DiscreteIntegrator  SampleTime=TimeStep  ICPrevOutput=DiscIntNeverNeededParam  ICPrevScaledInput=DiscIntNeverNeededParam
  Ground : Ground
  Ground1 : Ground
  Mux1 : Mux  Inputs=[3 3]
  Mux2 : Mux  Inputs=3
  Mux3 : Mux  Inputs=3
  Mux4 : Mux  Inputs=3
  Mux5 : Mux  Inputs=[3 3]
  Mux6 : Mux  Inputs=[6 3]
  Switch : Switch  Threshold=0.5
  Transformation_BODY2GLOBAL : PreScanSimulinkLibrary/Simple_Vehicle_Dynamics/Chassis/MuxDynState/Transformation_BODY2GLOBAL  SystemSampleTime=-1
  mux3 : Mux  Inputs=3
  state : Outport
  wires:
  Constant/1 -> Switch/2
  Mux6/1 -> state/1
  Mux4/1 -> Mux1/1
  Mux5/1 -> Switch/3
  Mux1/1 -> Switch/1
  Switch/1 -> Mux6/1  "PosVeh wrt  GLOB"
  P [rad]/1 -> Mux3/2
  R [rad]/1 -> Mux3/1
  dz [m/s]/1 -> Mux2/3
  dy [m/s]/1 -> Mux2/2
  dx [m/s]/1 -> Mux2/1
  z [m]/1 -> Mux4/3
  y [m]/1 -> Mux4/2
  x [m]/1 -> Mux4/1
  Ground1/1 -> mux3/2  "pitch"
  Y [rad]/1 -> mux3/3, Mux3/3  "yaw"
  Mux3/1 -> Mux6/2
  Transformation_BODY2GLOBAL/1 -> Discrete-Time Integrator/1, Mux5/2  "VelVEH wrt  GLOB"
  mux3/1 -> Transformation_BODY2GLOBAL/2
  Ground/1 -> mux3/1  "roll"
  Mux2/1 -> Mux1/2, Transformation_BODY2GLOBAL/1
  Discrete-Time Integrator/1 -> Mux5/1  "PosVEH wrt  GLOB"

== system Demo_IntelligentTrafficSigns_cs/CameraTrolley/SELF_Demux/compas converter ==
  rotz [rad] : Inport
  cart2compas : Subsystem
  rad2deg : Gain  Gain=180/pi
  heading [deg] : Outport
  wires:
  cart2compas/1(heading [deg]) -> heading [deg]/1
  rotz [rad]/1 -> rad2deg/1
  rad2deg/1 -> cart2compas/1(Angle [deg])

== system Demo_IntelligentTrafficSigns_cs/VISUWheels_BMW_X5_1/conv_RotWheelFL/RotMat2AxisAngle ==
  RotMat_Visu : Inport
  Data Type Conversion : DataTypeConversion
  Divide : Product  Inputs=*/
  Gain : Gain  Gain=2
  Mux : Mux  Inputs=3
  Mux1 : Mux  Inputs=2
  Saturation1 : Saturate  UpperLimit=3-0.001  LowerLimit=-1+0.001
  Trigonometric Function : Trigonometry
  U1 : Fcn  Expr=u[6]-u[8]
  U2 : Fcn  Expr=u[7]-u[3]
  U3 : Fcn  Expr=u[2]-u[4]
  U_Phi : Fcn  Expr=u[1]+u[5]+u[9]
  U_Phi1 : Fcn  Expr=acos((u(1)-1)/2)
  U_Phi2 : Fcn  Expr=acos((u(1)-1)/2)
  Angles_Visu : Outport
  wires:
  U_Phi2/1 -> Trigonometric Function/1
  Saturation1/1 -> U_Phi2/1
  U_Phi1/1 -> Data Type Conversion/1
  U_Phi/1 -> Saturation1/1, U_Phi1/1
  Divide/1 -> Mux1/1
  Mux1/1 -> Angles_Visu/1
  Gain/1 -> Divide/2
  Mux/1 -> Divide/1
  U3/1 -> Mux/3
  U2/1 -> Mux/2
  U1/1 -> Mux/1
  Trigonometric Function/1 -> Gain/1
  RotMat_Visu/1 -> U_Phi/1, U3/1, U2/1, U1/1
  Data Type Conversion/1 -> Mux1/2

== system Demo_IntelligentTrafficSigns_cs/VISUWheels_BMW_X5_1/conv_RotWheelFR/RotMat2AxisAngle ==
  RotMat_Visu : Inport
  Data Type Conversion : DataTypeConversion
  Divide : Product  Inputs=*/
  Gain : Gain  Gain=2
  Mux : Mux  Inputs=3
  Mux1 : Mux  Inputs=2
  Saturation1 : Saturate  UpperLimit=3-0.001  LowerLimit=-1+0.001
  Trigonometric Function : Trigonometry
  U1 : Fcn  Expr=u[6]-u[8]
  U2 : Fcn  Expr=u[7]-u[3]
  U3 : Fcn  Expr=u[2]-u[4]
  U_Phi : Fcn  Expr=u[1]+u[5]+u[9]
  U_Phi1 : Fcn  Expr=acos((u(1)-1)/2)
  U_Phi2 : Fcn  Expr=acos((u(1)-1)/2)
  Angles_Visu : Outport
  wires:
  Data Type Conversion/1 -> Mux1/2
  RotMat_Visu/1 -> U1/1, U2/1, U3/1, U_Phi/1
  Trigonometric Function/1 -> Gain/1
  U1/1 -> Mux/1
  U2/1 -> Mux/2
  U3/1 -> Mux/3
  Mux/1 -> Divide/1
  Gain/1 -> Divide/2
  Mux1/1 -> Angles_Visu/1
  Divide/1 -> Mux1/1
  U_Phi/1 -> U_Phi1/1, Saturation1/1
  U_Phi1/1 -> Data Type Conversion/1
  Saturation1/1 -> U_Phi2/1
  U_Phi2/1 -> Trigonometric Function/1

== system Demo_IntelligentTrafficSigns_cs/VISUWheels_BMW_X5_1/conv_RotWheelRL/RotMat2AxisAngle ==
  RotMat_Visu : Inport
  Data Type Conversion : DataTypeConversion
  Divide : Product  Inputs=*/
  Gain : Gain  Gain=2
  Mux : Mux  Inputs=3
  Mux1 : Mux  Inputs=2
  Saturation1 : Saturate  UpperLimit=3-0.001  LowerLimit=-1+0.001
  Trigonometric Function : Trigonometry
  U1 : Fcn  Expr=u[6]-u[8]
  U2 : Fcn  Expr=u[7]-u[3]
  U3 : Fcn  Expr=u[2]-u[4]
  U_Phi : Fcn  Expr=u[1]+u[5]+u[9]
  U_Phi1 : Fcn  Expr=acos((u(1)-1)/2)
  U_Phi2 : Fcn  Expr=acos((u(1)-1)/2)
  Angles_Visu : Outport
  wires:
  Data Type Conversion/1 -> Mux1/2
  RotMat_Visu/1 -> U1/1, U2/1, U3/1, U_Phi/1
  Trigonometric Function/1 -> Gain/1
  U1/1 -> Mux/1
  U2/1 -> Mux/2
  U3/1 -> Mux/3
  Mux/1 -> Divide/1
  Gain/1 -> Divide/2
  Mux1/1 -> Angles_Visu/1
  Divide/1 -> Mux1/1
  U_Phi/1 -> U_Phi1/1, Saturation1/1
  U_Phi1/1 -> Data Type Conversion/1
  Saturation1/1 -> U_Phi2/1
  U_Phi2/1 -> Trigonometric Function/1

== system Demo_IntelligentTrafficSigns_cs/VISUWheels_BMW_X5_1/conv_RotWheelRR/RotMat2AxisAngle ==
  RotMat_Visu : Inport
  Data Type Conversion : DataTypeConversion
  Divide : Product  Inputs=*/
  Gain : Gain  Gain=2
  Mux : Mux  Inputs=3
  Mux1 : Mux  Inputs=2
  Saturation1 : Saturate  UpperLimit=3-0.001  LowerLimit=-1+0.001
  Trigonometric Function : Trigonometry
  U1 : Fcn  Expr=u[6]-u[8]
  U2 : Fcn  Expr=u[7]-u[3]
  U3 : Fcn  Expr=u[2]-u[4]
  U_Phi : Fcn  Expr=u[1]+u[5]+u[9]
  U_Phi1 : Fcn  Expr=acos((u(1)-1)/2)
  U_Phi2 : Fcn  Expr=acos((u(1)-1)/2)
  Angles_Visu : Outport
  wires:
  Data Type Conversion/1 -> Mux1/2
  RotMat_Visu/1 -> U1/1, U2/1, U3/1, U_Phi/1
  Trigonometric Function/1 -> Gain/1
  U1/1 -> Mux/1
  U2/1 -> Mux/2
  U3/1 -> Mux/3
  Mux/1 -> Divide/1
  Gain/1 -> Divide/2
  Mux1/1 -> Angles_Visu/1
  Divide/1 -> Mux1/1
  U_Phi/1 -> U_Phi1/1, Saturation1/1
  U_Phi1/1 -> Data Type Conversion/1
  Saturation1/1 -> U_Phi2/1
  U_Phi2/1 -> Trigonometric Function/1

== system Demo_IntelligentTrafficSigns_cs/VISUWheels_BMW_X5_1/conv_TransWheelFL/pos_ini_Visu ==
  Constant : Constant  Value=x_ini_VRML
  Constant1 : Constant  Value=y_ini_VRML
  Constant2 : Constant  Value=z_ini_VRML
  Mux1 : Mux  Inputs=3
  pos_ini_Visu : Outport
  wires:
  Constant2/1 -> Mux1/3
  Constant1/1 -> Mux1/2
  Mux1/1 -> pos_ini_Visu/1
  Constant/1 -> Mux1/1

== system Demo_IntelligentTrafficSigns_cs/VISUWheels_BMW_X5_1/conv_TransWheelFL/transISO2Visu ==
  transISO : Inport
  Demux : Demux  Outputs=3
  trans_x_Visu : Outport
  trans_y_Visu : Outport  Port=2
  trans_z_Visu : Outport  Port=3
  wires:
  Demux/3 -> trans_z_Visu/1
  transISO/1 -> Demux/1
  Demux/1 -> trans_x_Visu/1
  Demux/2 -> trans_y_Visu/1  "rot_y"

== system Demo_IntelligentTrafficSigns_cs/VISUWheels_BMW_X5_1/conv_TransWheelFR/pos_ini_Visu ==
  Constant : Constant  Value=x_ini_VRML
  Constant1 : Constant  Value=y_ini_VRML
  Constant2 : Constant  Value=z_ini_VRML
  Mux1 : Mux  Inputs=3
  pos_ini_Visu : Outport
  wires:
  Constant/1 -> Mux1/1
  Mux1/1 -> pos_ini_Visu/1
  Constant1/1 -> Mux1/2
  Constant2/1 -> Mux1/3

== system Demo_IntelligentTrafficSigns_cs/VISUWheels_BMW_X5_1/conv_TransWheelFR/transISO2Visu ==
  transISO : Inport
  Demux : Demux  Outputs=3
  trans_x_Visu : Outport
  trans_y_Visu : Outport  Port=2
  trans_z_Visu : Outport  Port=3
  wires:
  Demux/3 -> trans_z_Visu/1
  transISO/1 -> Demux/1
  Demux/1 -> trans_x_Visu/1
  Demux/2 -> trans_y_Visu/1  "rot_y"

== system Demo_IntelligentTrafficSigns_cs/VISUWheels_BMW_X5_1/conv_TransWheelRL/pos_ini_Visu ==
  Constant : Constant  Value=x_ini_VRML
  Constant1 : Constant  Value=y_ini_VRML
  Constant2 : Constant  Value=z_ini_VRML
  Mux1 : Mux  Inputs=3
  pos_ini_Visu : Outport
  wires:
  Constant/1 -> Mux1/1
  Mux1/1 -> pos_ini_Visu/1
  Constant1/1 -> Mux1/2
  Constant2/1 -> Mux1/3

== system Demo_IntelligentTrafficSigns_cs/VISUWheels_BMW_X5_1/conv_TransWheelRL/transISO2Visu ==
  transISO : Inport
  Demux : Demux  Outputs=3
  trans_x_Visu : Outport
  trans_y_Visu : Outport  Port=2
  trans_z_Visu : Outport  Port=3
  wires:
  Demux/3 -> trans_z_Visu/1
  transISO/1 -> Demux/1
  Demux/1 -> trans_x_Visu/1
  Demux/2 -> trans_y_Visu/1  "rot_y"

== system Demo_IntelligentTrafficSigns_cs/VISUWheels_BMW_X5_1/conv_TransWheelRR/pos_ini_Visu ==
  Constant : Constant  Value=x_ini_VRML
  Constant1 : Constant  Value=y_ini_VRML
  Constant2 : Constant  Value=z_ini_VRML
  Mux1 : Mux  Inputs=3
  pos_ini_Visu : Outport
  wires:
  Constant/1 -> Mux1/1
  Mux1/1 -> pos_ini_Visu/1
  Constant1/1 -> Mux1/2
  Constant2/1 -> Mux1/3

== system Demo_IntelligentTrafficSigns_cs/VISUWheels_BMW_X5_1/conv_TransWheelRR/transISO2Visu ==
  transISO : Inport
  Demux : Demux  Outputs=3
  trans_x_Visu : Outport
  trans_y_Visu : Outport  Port=2
  trans_z_Visu : Outport  Port=3
  wires:
  Demux/3 -> trans_z_Visu/1
  transISO/1 -> Demux/1
  Demux/1 -> trans_x_Visu/1
  Demux/2 -> trans_y_Visu/1  "rot_y"

== system Demo_IntelligentTrafficSigns_cs/BMW_X5_1/Car_Rx_1_Demux/compas converter/cart2compas ==
  Angle [deg] : Inport
  Constant : Constant  Value=90
  Constant1 : Constant  Value=360
  Gain : Gain  Gain=1/360
  Gain1 : Gain  Gain=360
  Gain2 : Gain  Gain=-1
  Rounding Function : Rounding
  Sum : Sum  Inputs=|-+
  Sum1 : Sum  Inputs=-+|
  Sum2 : Sum  Inputs=++|
  heading [deg] : Outport
  wires:
  Gain/1 -> Rounding Function/1
  Rounding Function/1 -> Gain1/1
  Gain1/1 -> Sum/1
  Angle [deg]/1 -> Sum1/2
  Sum/1 -> Gain2/1
  Sum1/1 -> Gain/1, Sum/2
  Constant/1 -> Sum1/1
  Gain2/1 -> Sum2/2
  Constant1/1 -> Sum2/1
  Sum2/1 -> heading [deg]/1

== system Demo_IntelligentTrafficSigns_cs/BMW_X5_1/INTERPRETATION ALGORITHM TrafficSign Intelligence/Convert and Sort Sensor Data/Sort Output Based on ID ==
  .. : Inport
  ids [-] : Inport  Port=2
  lats [deg] : Inport  Port=3
  longs [deg] : Inport  Port=4
  xs [m] : Inport  Port=5
  ys [m] : Inport  Port=6
  headings [deg] : Inport  Port=7
  velocity_limits [km/h] : Inport  Port=8
  notifications [-] : Inport  Port=9
  R_curves [m] : Inport  Port=10
  Embedded MATLAB Function : Stateflow chart  TreatAsAtomicUnit=on
  Selector{1..6,8..10} (9 alike) : Selector  InputPortWidth=portdim  IndexOptions=Index vector (port)  Indices=[1 3]  OutputSizes=1
  Selector7 : Selector  InputPortWidth=portdim  IndexOptions=Index vector (dialog)  Indices=OutputOrder  OutputSizes=1
  ids  [-] : Outport
  lats  [deg] : Outport  Port=2
  longs  [deg] : Outport  Port=3
  headings  [deg] : Outport  Port=4
  xs  [m] : Outport  Port=5
  ys  [m] : Outport  Port=6
  velocity_limits  [km/h] : Outport  Port=7
  notifications  [-] : Outport  Port=8
  R_curves  [m] : Outport  Port=9
  wires:
  R_curves [m]/1 -> Selector10/1
  Selector10/1 -> R_curves  [m]/1
  notifications [-]/1 -> Selector9/1
  Selector9/1 -> notifications  [-]/1
  Selector8/1 -> ys  [m]/1
  ys [m]/1 -> Selector8/1
  Selector6/1 -> xs  [m]/1
  xs [m]/1 -> Selector6/1
  Selector5/1 -> velocity_limits  [km/h]/1
  velocity_limits [km/h]/1 -> Selector5/1
  headings [deg]/1 -> Selector4/1
  Selector4/1 -> headings  [deg]/1
  Selector3/1 -> longs  [deg]/1
  longs [deg]/1 -> Selector3/1
  lats [deg]/1 -> Selector2/1
  Selector2/1 -> lats  [deg]/1
  Selector7/1 -> Selector6/2, Selector8/2, Selector10/2, Selector9/2, Selector5/2, Selector4/2, Selector3/2, Selector2/2, Selector1/2
  ../1 -> Embedded MATLAB Function/1(u)
  Selector1/1 -> ids  [-]/1
  ids [-]/1 -> Selector1/1
  Embedded MATLAB Function/1(y) -> Selector7/1

== system Demo_IntelligentTrafficSigns_cs/BMW_X5_1/INTERPRETATION ALGORITHM TrafficSign Intelligence/NOTIFICATION  UPCOMING TRAFFIC SIGN/HOLD logic ==
  In : Inport
  Memory : Memory  X0=80
  Switch : Switch  Criteria=u2 > Threshold
  Out : Outport
  wires:
  In/1 -> Switch/1, Switch/2
  Memory/1 -> Switch/3
  Switch/1 -> Out/1, Memory/1

== system Demo_IntelligentTrafficSigns_cs/BMW_X5_1/INTERPRETATION ALGORITHM TrafficSign Intelligence/NOTIFICATION  UPCOMING TRAFFIC SIGN/Retrieve upcoming prescribed velocity ==
  notifications [-] : Inport
  velocity_limits [km/h] : Inport  Port=2
  Product1 : Product  RndMeth=Zero
  Sum1 : Sum  Inputs=+
  upcoming velocity_limit  [km/h] : Outport
  wires:
  notifications [-]/1 -> Product1/1
  Product1/1 -> Sum1/1
  velocity_limits [km/h]/1 -> Product1/2
  Sum1/1 -> upcoming velocity_limit  [km/h]/1

== system Demo_IntelligentTrafficSigns_cs/BMW_X5_1/INTERPRETATION ALGORITHM TrafficSign Intelligence/RECOMMENDED  SAFE SPEED IN CURVE/Curve warning mechanism define recommende speed based on host_velocity and curve radius ==
  velocity [m/s] : Inport
  R_curve [m] : Inport  Port=2
  allowed ay limit [m/s2] : Inport  Port=3
  Data Type Conversion : DataTypeConversion
  Divide : Product  Inputs=/*
  Divide1 : Product  Inputs=*/
  Divide2 : Product
  Math Function : Math  Operator=magnitude^2  SaturateOnIntegerOverflow=on
  Math Function1 : Math  Operator=sqrt  SaturateOnIntegerOverflow=on
  Relational Operator : RelationalOperator  OutDataTypeStr=boolean
  v_recommended [m/s] : Outport
  warning [-] : Outport  Port=2
  ay_limit [m/s2] : Outport  Port=3
  R_safe [m] : Outport  Port=4
  wires:
  Math Function1/1 -> v_recommended [m/s]/1
  Divide2/1 -> Math Function1/1
  Data Type Conversion/1 -> warning [-]/1
  Relational Operator/1 -> Data Type Conversion/1
  Math Function/1 -> Divide1/1, Divide/2
  Divide1/1 -> R_safe [m]/1
  R_curve [m]/1 -> Divide2/2, Divide/1
  velocity [m/s]/1 -> Math Function/1
  Divide/1 -> Relational Operator/1, ay_limit [m/s2]/1
  allowed ay limit [m/s2]/1 -> Divide2/1, Relational Operator/2, Divide1/2

== system Demo_IntelligentTrafficSigns_cs/BMW_X5_1/INTERPRETATION ALGORITHM TrafficSign Intelligence/RECOMMENDED  SAFE SPEED IN CURVE/HOLD logic1 ==
  In : Inport
  Memory : Memory
  Switch : Switch  Criteria=u2 > Threshold
  Out : Outport
  wires:
  Switch/1 -> Memory/1, Out/1
  Memory/1 -> Switch/3
  In/1 -> Switch/2, Switch/1

== system Demo_IntelligentTrafficSigns_cs/BMW_X5_1/INTERPRETATION ALGORITHM TrafficSign Intelligence/RECOMMENDED  SAFE SPEED IN CURVE/Replace zero radius (no warning) with infinite radius ==
  R_curve [m] : Inport
  Constant4 : Constant  Value=0
  Data Type Conversion : DataTypeConversion
  Gain : Gain  Gain=1e6
  Relational Operator1 : RelationalOperator  Operator===  OutDataTypeStr=boolean
  Sum : Sum  Inputs=|++
  filt_R_curve [m] : Outport
  wires:
  Gain/1 -> Sum/2
  Data Type Conversion/1 -> Gain/1
  Relational Operator1/1 -> Data Type Conversion/1
  Constant4/1 -> Relational Operator1/2
  R_curve [m]/1 -> Sum/1, Relational Operator1/1
  Sum/1 -> filt_R_curve [m]/1

== system Demo_IntelligentTrafficSigns_cs/BMW_X5_1/INTERPRETATION ALGORITHM TrafficSign Intelligence/RECOMMENDED  SAFE SPEED IN CURVE/Retrieve radius of upcoming curve ==
  notifications [-] : Inport
  R_curves [m] : Inport  Port=2
  Product1 : Product  RndMeth=Zero
  Sum1 : Sum  Inputs=+
  R_curves   [m] : Outport
  wires:
  notifications [-]/1 -> Product1/1
  Product1/1 -> Sum1/1
  R_curves [m]/1 -> Product1/2
  Sum1/1 -> R_curves   [m]/1

== system Demo_IntelligentTrafficSigns_cs/BMW_X5_1/INTERPRETATION ALGORITHM TrafficSign Intelligence/SPEED LIMIT/HOLD logic ==
  In : Inport
  Memory : Memory  X0=80
  Switch : Switch  Criteria=u2 > Threshold
  Out : Outport
  wires:
  In/1 -> Switch/1, Switch/2
  Memory/1 -> Switch/3
  Switch/1 -> Out/1, Memory/1

== system Demo_IntelligentTrafficSigns_cs/BMW_X5_1/INTERPRETATION ALGORITHM TrafficSign Intelligence/SPEED LIMIT/Retrieve Prescribed Velocity ==
  permissions [-] : Inport
  velocity_limits [km/h] : Inport  Port=2
  Product1 : Product  RndMeth=Zero
  Sum1 : Sum  Inputs=+
  velocity_limit [km/h] : Outport
  wires:
  permissions [-]/1 -> Product1/1
  Product1/1 -> Sum1/1
  velocity_limits [km/h]/1 -> Product1/2
  Sum1/1 -> velocity_limit [km/h]/1

== system Demo_IntelligentTrafficSigns_cs/BMW_X5_1/INTERPRETATION ALGORITHM TrafficSign Intelligence/TRAFFIC SIGN DETECTION based on relative  position and orientation/TRAFFIC SIGN DETECTION: based on Heading ==
  heading_host [deg] : Inport
  headings [deg] : Inport  Port=2
  Abs : Abs
  Heading : Subsystem  Description=-270 <= u <= -90  mask(Checks_SRange){max=180+45, max_included=on, min=180-45, min_included=on}
      mask prompts: max "Upper bound:"; max_included "Inclusive upper bound"; min "Lower bound:"; min_included "Inclusive lower bound"
      mask help: Assert that the input signal lies between a static lower and upper bound or optionally equals either bound.
      mask Display: image(imread('Checks_SRange.bmp','bmp'),'center')
  Sum1 : Sum  Inputs=+-
  relevance [-] : Outport
  wires:
  headings [deg]/1 -> Sum1/2
  heading_host [deg]/1 -> Sum1/1
  Abs/1 -> Heading/1(u)
  Heading/1(Out1) -> relevance [-]/1  "lim_heading [deg]"
  Sum1/1 -> Abs/1  "rel_heading [deg]"

== system Demo_IntelligentTrafficSigns_cs/BMW_X5_1/INTERPRETATION ALGORITHM TrafficSign Intelligence/TRAFFIC SIGN DETECTION based on relative  position and orientation/TRAFFIC SIGN DETECTION: based on Position ==
  x_host [m] : Inport
  y_host [m] : Inport  Port=2
  xs [m] : Inport  Port=3
  ys [m] : Inport  Port=4
  heading_host [deg] : Inport  Port=5
  Demux : Demux  Outputs=2
  Demux1 : Demux  Outputs=2
  Mux : Mux  Inputs=2
  Product1 : Product  RndMeth=Zero
  Product3 : Product  RndMeth=Zero
  Sum4 : Sum  Inputs=+-
  Sum5 : Sum  Inputs=+-
  Transformation_GLOBAL2LOC2 : Subsystem
  dx_Intervene : Subsystem  Description=-270 <= u <= -90  mask(Checks_SRange){max=0, max_included=on, min=-1*1*50/3.6, min_included=on}
      mask prompts: max "Upper bound:"; max_included "Inclusive upper bound"; min "Lower bound:"; min_included "Inclusive lower bound"
      mask help: Assert that the input signal lies between a static lower and upper bound or optionally equals either bound.
      mask Display: image(imread('Checks_SRange.bmp','bmp'),'center')
  dx_notification : Subsystem  Description=-270 <= u <= -90  mask(Checks_SRange){max=0, max_included=on, min=-1*3*50/3.6, min_included=on}
      mask prompts: max "Upper bound:"; max_included "Inclusive upper bound"; min "Lower bound:"; min_included "Inclusive lower bound"
      mask help: Assert that the input signal lies between a static lower and upper bound or optionally equals either bound.
      mask Display: image(imread('Checks_SRange.bmp','bmp'),'center')
  dy_Intervene : Subsystem  Description=-270 <= u <= -90  mask(Checks_SRange){max=5, max_included=on, min=-2, min_included=on}
      mask prompts: max "Upper bound:"; max_included "Inclusive upper bound"; min "Lower bound:"; min_included "Inclusive lower bound"
      mask help: Assert that the input signal lies between a static lower and upper bound or optionally equals either bound.
      mask Display: image(imread('Checks_SRange.bmp','bmp'),'center')
  dy_notification : Subsystem  Description=-270 <= u <= -90  mask(Checks_SRange){max=5, max_included=on, min=-2, min_included=on}
      mask prompts: max "Upper bound:"; max_included "Inclusive upper bound"; min "Lower bound:"; min_included "Inclusive lower bound"
      mask help: Assert that the input signal lies between a static lower and upper bound or optionally equals either bound.
      mask Display: image(imread('Checks_SRange.bmp','bmp'),'center')
  permissions [-] : Outport
  notifications [-] : Outport  Port=2
  wires:
  xs [m]/1 -> Sum5/2
  ys [m]/1 -> Sum4/2
  Sum4/1 -> Transformation_GLOBAL2LOC2/2(ys_GLOB [m])  "vector dy global [m]"
  Sum5/1 -> Transformation_GLOBAL2LOC2/1(xs_GLOB [m])  "vector dx global [m]"
  heading_host [deg]/1 -> Transformation_GLOBAL2LOC2/3(angle_GLOBwrtLOC headings [deg])  "heading"
  x_host [m]/1 -> Sum5/1
  y_host [m]/1 -> Sum4/1
  dx_notification/1(Out1) -> Product3/1  "dx_limit"
  dy_notification/1(Out1) -> Product3/2  "dy_limit"
  Transformation_GLOBAL2LOC2/1(xs_LOC [m]) -> Mux/1
  Transformation_GLOBAL2LOC2/2(ys_LOC [m]) -> Mux/2
  Product3/1 -> notifications [-]/1
  dx_Intervene/1(Out1) -> Product1/1  "dx_limit"
  dy_Intervene/1(Out1) -> Product1/2  "dy_limit"
  Demux/1 -> dx_notification/1(u)
  Demux/2 -> dy_notification/1(u)
  Product1/1 -> permissions [-]/1
  Demux1/1 -> dx_Intervene/1(u)
  Demux1/2 -> dy_Intervene/1(u)
  Mux/1 -> Demux/1, Demux1/1
